simulink model "FlightSim"  (R2023a)
settings: Solver=VariableStepAuto  StartTime=0.0  StopTime=20  RelTol=1e-3
model workspace (MAT data in the model file):
  polyCoefficient = [1 1 1]
values from neighbouring files (symbols this model uses):
  airDensity = 1.2250    (= 1.225)    [FlightSimSetup.m]
  airKinematicViscosity = 1.48*10^-5    (= 1.48e-05)    [FlightSimSetup.m]
  AOA = deg2rad(0)    [FlightSimSetup.m]
  axialDistanceMACCGx = abs(finMACLeadingEdgeLocation) + finLeadingEdgeCGxlength    [finParameters.m]
  bodyDiameter = 2/100    (= 0.02)    [FlightSimSetup.m]
  bodyLength = 20/100    (= 0.2)    [FlightSimSetup.m]
  bodyLongitudinalInertia = bodyMass*( ((bodyDiameter/2).^2)/4 + ((bodyLength).^2)/12)    [InertiaParameters.m]
  bodyMass = 12/1000    (= 0.012)    [FlightSimSetup.m]
  bodyPlanformArea = bodyDiameter*bodyLength    (= 0.004)    [FlightSimSetup.m]
  bodyRoughness = 60*10^-6    (= 6e-05)    [FlightSimSetup.m]
  casingLongitudinalInertia = motorCasingMass/12*(3*(motorOuterRadius.^2+motorInnerRadius.^2)+motorLength.^2)    [InertiaParameters.m]
  cYaw = 0    [FlightSimSetup.m]
  dIdt = zeros(3)    [InertiaParameters.m]
  finArea = (finRootChord+finTailChord)*finSpan/2    [finParameters.m]
  finCantAngle = deg2rad(0)    [FlightSimSetup.m]
  finCentroidAxial = finSpan/3 * ( (finRootChord + 2*finTailChord)/(finRootChord + finTailChord) ) - backOffset    [finParameters.m]
  finCount = 4    [FlightSimSetup.m]
  finFrontSweepAngle = acos(dot(frontSweepAngleRefVector1, frontSweepAngleRefVector2) / (norm(frontSweepAngleRefVector1) * norm(frontSweepAngleRefVector2)))    [finParameters.m]
  finLeadingEdgeNoseConeDistance = bodyLength + noseConeHeight - finRootChord + backOffset    [finParameters.m]
  finLongitudinalInertia = finLongitudinalInertia * singleFinMass * finCount    [InertiaParameters.m]
  finMACLeadingEdgeLocation = abs( integral(finMACLeadingEdgeLocationFunction, 0, finSpan)/finArea )    [finParameters.m]
  finMACLength = integral(chordLengthFunction, 0, finSpan)/finArea    [finParameters.m]
  finMACNoseconeAxialDistance = finLeadingEdgeNoseConeDistance + finMACLeadingEdgeLocation+ 1/2*finMACLength    [finParameters.m]
  finMidChordSweepAngle = acos(dot(midChordAngleRefVector1, midChordAngleRefVector2) / (norm(midChordAngleRefVector1) * norm(midChordAngleRefVector2)))    [finParameters.m]
  finProfileType = 3    [FlightSimSetup.m]
  finRootChord = abs(P_frontRoot(2)-P_backRoot(2))    [finParameters.m]
  finSpan = abs(P_frontTrail(1)- P_frontRoot(1))    [finParameters.m]
  finTailChord = abs(P_frontTrail(2)-P_backTrail(2))    [finParameters.m]
  finThickness = 0.15/100    (= 0.0015)    [FlightSimSetup.m]
  finX_t = P_frontRoot(2) - P_frontTrail(2)    [finParameters.m]
  launchLugInnerDiameter = 0    [FlightSimSetup.m]
  launchLugLength = 0    [FlightSimSetup.m]
  launchLugOuterDiameter = 0    [FlightSimSetup.m]
  machNum = 0    [FlightSimSetup.m]
  motorCasingMass = motorInitialTotalMass - motorInitialPropMass    (= 0.071)    [FlightSimSetup.m]
  motorInitialPropMass = 83/1000    (= 0.083)    [FlightSimSetup.m]
  motorInnerRadius = motorOuterRadius - casingThickness    [InertiaParameters.m]
  motorLength = 156/1000    (= 0.156)    [FlightSimSetup.m]
  motorTotalImpulse = 129.9    [FlightSimSetup.m]
  noseConeHeight = 6/100    (= 0.06)    [FlightSimSetup.m]
  noseConeJoinAngle = pi/2 - atan(noseConeHeight/(bodyDiameter/2))    [FlightSimSetup.m]
  noseConeLongitudinalInertia = noseConeLongitudinalInertia*noseConeMass    [noseConeParameters.m]
  noseConeMass = 5/1000    (= 0.005)    [FlightSimSetup.m]
  noseConePlanformArea = (1/2)*bodyDiameter* noseConeHeight    [noseConeParameters.m]
  noseConeType = 8    [FlightSimSetup.m]
  noseConeVolume = (1/3)*noseConeHeight*(bodyDiameter/2)^2*pi    [noseConeParameters.m]
  noseConeWettedArea = pi*sqrt( (bodyDiameter/2)^2 + noseConeHeight^2)*bodyDiameter/2    [noseConeParameters.m]
  pitchCenterX = 0    [FlightSimSetup.m]
  pitchRate = 0    [FlightSimSetup.m]
  radialMACPosition = finMACSpanwisePosition + bodyDiameter/2    [finParameters.m]
  refArea = bodyDiameter^2*pi/4    (= 0.000314159265359)    [FlightSimSetup.m]
  rocketLength = (20+6)/100    (= 0.26)    [FlightSimSetup.m]
  rocketNonMotorMass = noseConeMass + bodyMass + totalFinMass    (= 0.021)    [FlightSimSetup.m]
  rocketRotationalInertia = bodyRotationalInertia + casingRotationalInertia + finRotationalInertia + noseConeLongitudinalInertia    [InertiaParameters.m]
  rocketVelocityMag = machNum*340.17    (= 0)    [FlightSimSetup.m]
  rollRate = 0    [FlightSimSetup.m]
  totalFinMass = singleFinMass * finCount    (= 0.004)    [FlightSimSetup.m]
  windAngle = 0    [FlightSimSetup.m]
  yawRate = 0    [FlightSimSetup.m]
libraries: shared6dof x1, aerolibanim x1, aerolibadyn x1, aerolibbdyn x1, sharedutil x1
(part 1 of 3)
settings Model: Creator=ericyang  LastModifiedBy=ericyang
settings DataIOCC: ExternalInput=[t,u]

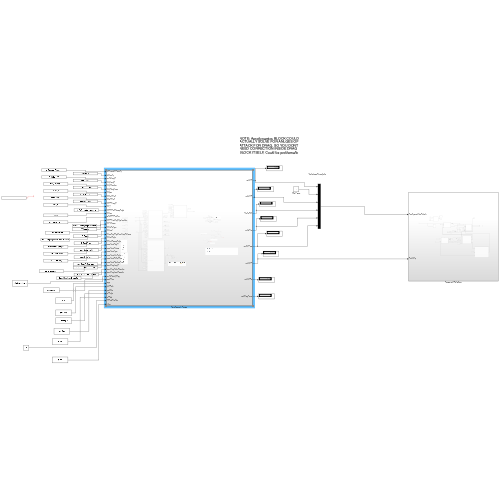
[thumbnail: Simulink's stored preview of the top-level diagram]
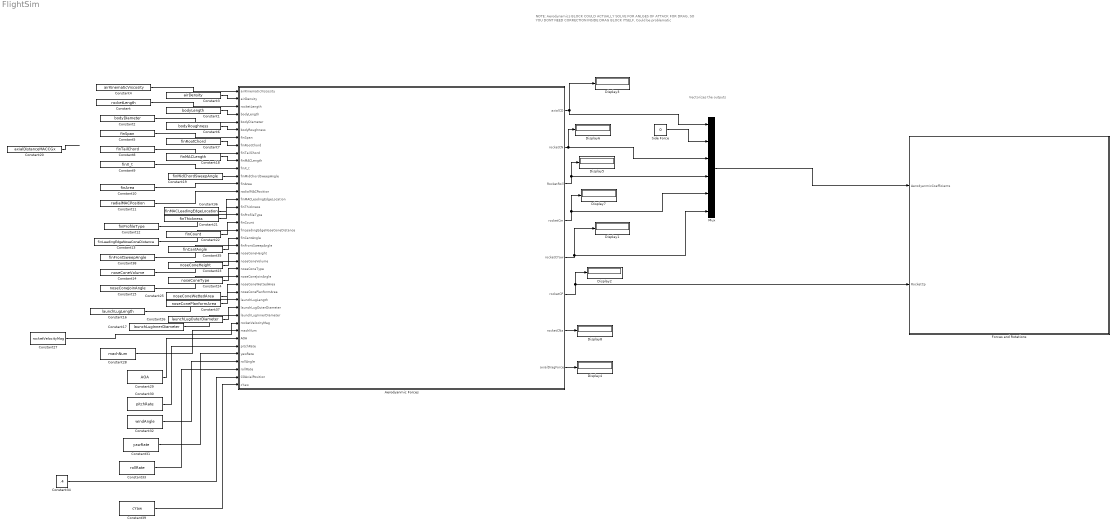
== system FlightSim ==
  note: NOTE: Aerodynamics BLOCK COULD ACTUALLY SOLVE FOR ANLGES OF ATTACK FOR DRAG, SO YOU DONT NEED CORRECTION INSIDE DRAG BLOCK ITSELF, Could be problematic
  note: Vectorizes the outputs
  Aerodyanmic Forces : Subsystem
  Constant : Constant  Value=rocketLength
  Constant1 : Constant  Value=bodyLength
  Constant10 : Constant  Value=finArea
  Constant11 : Constant  Value=radialMACPosition
  Constant12 : Constant  Value=finProfileType
  Constant13 : Constant  Value=finLeadingEdgeNoseConeDistance
  Constant14 : Constant  Value=noseConeVolume
  Constant15 : Constant  Value=noseConeJoinAngle
  Constant16 : Constant  Value=launchLugLength
  Constant17 : Constant  Value=launchLugInnerDiameter
  Constant18 : Constant  Value=finMACLength
  Constant19 : Constant  Value=finMidChordSweepAngle
  Constant2 : Constant  Value=bodyDiameter
  Constant20 : Constant  Value=axialDistanceMACCGx
  Constant21 : Constant  Value=finThickness
  Constant22 : Constant  Value=finCount
  Constant23 : Constant  Value=noseConeHeight
  Constant24 : Constant  Value=noseConeType
  Constant25 : Constant  Value=noseConeWettedArea
  Constant26 : Constant  Value=launchLugOuterDiameter
  Constant27 : Constant  Value=rocketVelocityMag
  Constant28 : Constant  Value=machNum
  Constant29 : Constant  Value=AOA
  Constant3 : Constant  Value=airDensity
  Constant30 : Constant  Value=pitchRate
  Constant31 : Constant  Value=yawRate
  Constant32 : Constant  Value=windAngle
  Constant33 : Constant  Value=rollRate
  Constant34 : Constant  Value=.4
  Constant35 : Constant  Value=finCantAngle
  Constant36 : Constant  Value=finMACLeadingEdgeLocation
  Constant37 : Constant  Value=noseConePlanformArea
  Constant38 : Constant  Value=finFrontSweepAngle
  Constant39 : Constant  Value=cYaw
  Constant4 : Constant  Value=airKinematicViscosity
  Constant5 : Constant  Value=finSpan
  Constant6 : Constant  Value=bodyRoughness
  Constant7 : Constant  Value=finRootChord
  Constant8 : Constant  Value=finTailChord
  Constant9 : Constant  Value=finX_t
  Display{1..8} (8 alike) : Display
  Forces and Rotations : Subsystem
  Mux : Mux  Inputs=6
  Side Force : Constant  Value=0
  wires:
  Constant/1 -> Aerodyanmic Forces/3(rocketLength)
  Constant1/1 -> Aerodyanmic Forces/4(bodyLength)
  Constant2/1 -> Aerodyanmic Forces/5(bodyDiameter)
  Constant4/1 -> Aerodyanmic Forces/1(airKinematicViscosity)
  Constant3/1 -> Aerodyanmic Forces/2(airDensity)
  Constant6/1 -> Aerodyanmic Forces/6(bodyRoughness)
  Constant5/1 -> Aerodyanmic Forces/7(finSpan)
  Constant7/1 -> Aerodyanmic Forces/8(finRootChord)
  Constant8/1 -> Aerodyanmic Forces/9(finTailChord)
  Constant9/1 -> Aerodyanmic Forces/11(finX_t)
  Constant10/1 -> Aerodyanmic Forces/13(finArea)
  Constant11/1 -> Aerodyanmic Forces/14(radialMACPosition)
  Constant12/1 -> Aerodyanmic Forces/17(finProfileType)
  Constant13/1 -> Aerodyanmic Forces/19(finLeadingEdgeNoseConeDistance)
  Constant14/1 -> Aerodyanmic Forces/23(noseConeVolume)
  Constant15/1 -> Aerodyanmic Forces/25(noseConeJoinAngle)
  Constant16/1 -> Aerodyanmic Forces/28(launchLugLength)
  Constant17/1 -> Aerodyanmic Forces/30(launchLugInnerDiameter)
  Constant18/1 -> Aerodyanmic Forces/10(finMACLength)
  Constant19/1 -> Aerodyanmic Forces/12(finMidChordSweepAngle)
  Constant20/1 -> ?
  Constant21/1 -> Aerodyanmic Forces/16(finThickness)
  Constant22/1 -> Aerodyanmic Forces/18(finCount)
  Constant23/1 -> Aerodyanmic Forces/22(noseConeHeight)
  Constant24/1 -> Aerodyanmic Forces/24(noseConeType)
  Constant25/1 -> Aerodyanmic Forces/26(noseConeWettedArea)
  Constant26/1 -> Aerodyanmic Forces/29(launchLugOuterDiameter)
  Constant27/1 -> Aerodyanmic Forces/31(rocketVelocityMag)
  Constant28/1 -> Aerodyanmic Forces/32(machNum)
  Constant29/1 -> Aerodyanmic Forces/33(AOA)
  Constant30/1 -> Aerodyanmic Forces/34(pitchRate)
  Constant31/1 -> Aerodyanmic Forces/35(yawRate)
  Constant32/1 -> Aerodyanmic Forces/36(rollAngle)
  Constant33/1 -> Aerodyanmic Forces/37(rollRate)
  Aerodyanmic Forces/6(rocketCP) -> Forces and Rotations/2(RocketCp), Display2/1
  Aerodyanmic Forces/1(axialCD) -> Display3/1, Mux/1
  Aerodyanmic Forces/8(axialDragForce) -> Display4/1
  Aerodyanmic Forces/3(RocketRoll) -> Mux/4, Display5/1
  Aerodyanmic Forces/2(rocketCN) -> Display6/1, Mux/3
  Aerodyanmic Forces/4(rocketCm) -> Mux/5, Display7/1
  Constant35/1 -> Aerodyanmic Forces/20(finCantAngle)
  Constant36/1 -> Aerodyanmic Forces/15(finMACLeadingEdgeLocation)
  Constant37/1 -> Aerodyanmic Forces/27(noseConePlanformArea)
  Constant38/1 -> Aerodyanmic Forces/21(finFrontSweepAngle)
  Constant39/1 -> Aerodyanmic Forces/39(cYaw)
  Aerodyanmic Forces/7(rocketCNa) -> Display8/1
  Side Force/1 -> Mux/2
  Aerodyanmic Forces/5(rocketCYaw) -> Mux/6, Display1/1
  Mux/1 -> Forces and Rotations/1(AerodyanmicCoefficients)
  Constant34/1 -> Aerodyanmic Forces/38(CGAxialPosition)

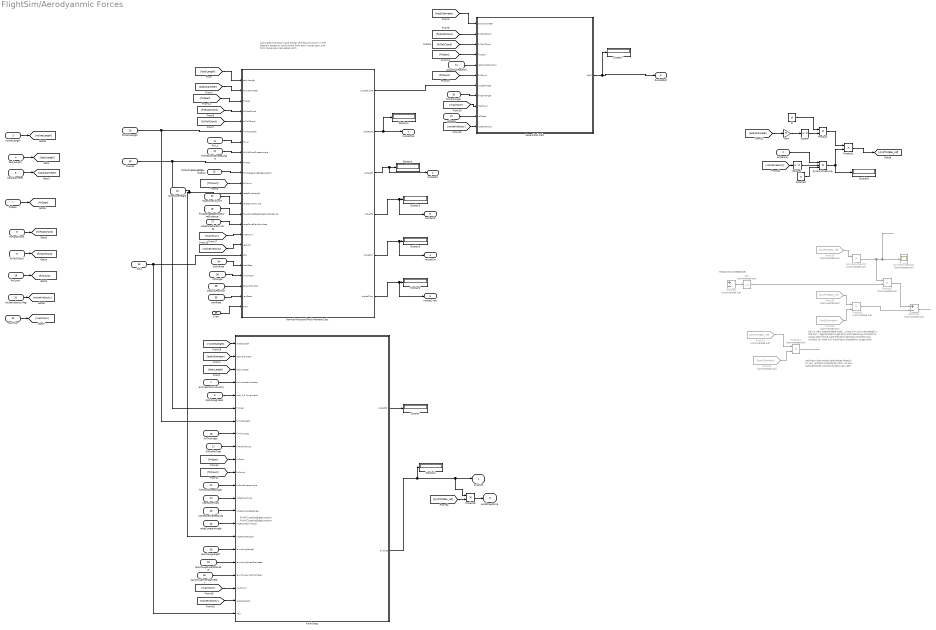
== system FlightSim/Aerodyanmic Forces ==
  note: finMACLeadingEdgeLocation
  note: finMACLeadingEdgeLocation
  note: Relative to NOSECONE
  note: nothing in the rocket contributes directly to yaw (without canards for now), so only outside forces would provide a yaw rate
  note: this is very questionable math... since Cm isn't calculated in the sim, i figured that to get form pitch damping moment to actual pitch force, take the pitch damping moment and multiply by what Cm would be multiplied by to get pitch
  note: calculates the total N and Center of Pressure shown in the diagram based on contriutions from each rocket part, and from those also calculates pitch
  airKinematicViscosity : Inport
  airDensity : Inport  Port=2
  rocketLength : Inport  Port=3
  bodyLength : Inport  Port=4
  bodyDiameter : Inport  Port=5
  bodyRoughness : Inport  Port=6
  finSpan : Inport  Port=7
  finRootChord : Inport  Port=8
  finTailChord : Inport  Port=9
  finMACLength : Inport  Port=10
  finX_t : Inport  Port=11
  finMidChordSweepAngle : Inport  Port=12
  finArea : Inport  Port=13
  radialMACPosition : Inport  Port=14
  finMACLeadingEdgeLocation : Inport  Port=15
  finThickness : Inport  Port=16
  finProfileType : Inport  Port=17
  finCount : Inport  Port=18
  finLeadingEdgeNoseConeDistance : Inport  Port=19
  finCantAngle : Inport  Port=20
  finFrontSweepAngle : Inport  Port=21
  noseConeHeight : Inport  Port=22
  noseConeVolume : Inport  Port=23
  noseConeType : Inport  Port=24
  noseConeJoinAngle : Inport  Port=25
  noseConeWettedArea : Inport  Port=26
  noseConePlanformArea : Inport  Port=27
  launchLugLength : Inport  Port=28
  launchLugOuterDiameter : Inport  Port=29
  launchLugInnerDiameter : Inport  Port=30
  rocketVelocityMag : Inport  Port=31
  machNum : Inport  Port=32
  AOA : Inport  Port=33
  pitchRate : Inport  Port=34
  yawRate : Inport  Port=35
  rollAngle : Inport  Port=36
  rollRate : Inport  Port=37
  CGAxialPosition : Inport  Port=38
  cYaw : Inport  Port=39
  Abs : Abs  Commented=on  SaturateOnIntegerOverflow=off
  Axial Drag : Subsystem
  Canard Roll Calc : Subsystem
  Constant : Constant  Value=.5
  Display{,1..8} (9 alike) : Display
  From23 : From  Commented=on  GotoTag=bodyDiameter
  From24 : From  Commented=on  GotoTag=bodyDiameter
  From28 : From  Commented=on  GotoTag=dynPTIMESA_ref
  From30 : From  Commented=on  GotoTag=dynPTIMESA_ref
  From31 : From  Commented=on  GotoTag=dynPTIMESA_ref
  Gain : Gain  Gain=.5
  Normal Force and Pitch Moment Calc : Subsystem
  Pi : Constant  Value=pi  OutDataTypeStr=Inherit: Inherit via back propagation
  Product : Product
  Product1 : Product
  Product2 : Product  Commented=on
  Product3 : Product  Commented=on  Inputs=3
  Product4 : Product  Commented=on  Inputs=3
  Product6 : Product
  Square : Math  Operator=square
  Square1 : Math  Operator=square
  Subtract : Sum  Commented=on  Inputs=+-
  Subtract1 : Sum  Commented=on  Inputs=+-
  dynamicPressure : Product  Inputs=3
  normalForceAtCP : Product  Commented=on
  normalForceScope : Scope  Commented=on
  axialCD : Outport
  rocketCN : Outport  Port=2
  RocketRoll : Outport  Port=3
  rocketCm : Outport  Port=4
  rocketCYaw : Outport  Port=5
  rocketCP : Outport  Port=6
  rocketCNa : Outport  Port=7
  axialDragForce : Outport  Port=8
  wires:
  finX_t/1 -> Normal Force and Pitch Moment Calc/7(finX_t)
  finMidChordSweepAngle/1 -> Normal Force and Pitch Moment Calc/8(finMidChordSweepAngle)
  finArea/1 -> Axial Drag/6(finArea), Normal Force and Pitch Moment Calc/9(finArea)
  finProfileType/1 -> Axial Drag/9(finProfileType)
  finThickness/1 -> Axial Drag/8(finThickness)
  radialMACPosition/1 -> Canard Roll Calc/5(radialMACPosition)
  finLeadingEdgeNoseConeDistance/1 -> Normal Force and Pitch Moment Calc/14(finLeadingEdgeNoseConeDistance)
  noseConeType/1 -> Axial Drag/13(noseConeType)
  noseConeWettedArea/1 -> Axial Drag/14(noseConeWettedArea)
  noseConeJoinAngle/1 -> Axial Drag/15(noseConeJoinAngle)
  noseConeVolume/1 -> Normal Force and Pitch Moment Calc/13(noseConeVolume)
  launchLugLength/1 -> Axial Drag/17(launchLugLength)
  launchLugOuterDiameter/1 -> Axial Drag/18(launchLugOuterDiameter)
  launchLugInnerDiameter/1 -> Axial Drag/19(launchLugInnerDiameter)
  rollRate/1 -> Canard Roll Calc/10(" rollRate")
  Normal Force and Pitch Moment Calc/1(singleFinCNa) -> Canard Roll Calc/7(singleFinCNa)
  bodyLength/1 -> [bodyLength]
  bodyDiameter/1 -> [bodyDiameter]
  finCount/1 -> [finCount]
  [bodyLength] -> Normal Force and Pitch Moment Calc/1(bodyLength)
  [bodyLength] -> Axial Drag/3(bodyLength)
  [bodyDiameter] -> Normal Force and Pitch Moment Calc/2(bodyDiameter)
  [bodyDiameter] -> Canard Roll Calc/1(bodyDiameter)
  [bodyDiameter] -> Axial Drag/2(bodyDiameter)
  airKinematicViscosity/1 -> Axial Drag/4(airKinematicViscocity)
  bodyRoughness/1 -> Axial Drag/5(bodyR_S (roughness))
  finRootChord/1 -> [finRootChord]
  finTailChord/1 -> [finTailChord]
  [finRootChord] -> Normal Force and Pitch Moment Calc/4(finRootChord)
  [finRootChord] -> Canard Roll Calc/2(finRootChord)
  [finTailChord] -> Normal Force and Pitch Moment Calc/5(finTailChord)
  [finTailChord] -> Canard Roll Calc/3(finTailChord)
  [finCount] -> Normal Force and Pitch Moment Calc/11(finCount)
  [finCount] -> Canard Roll Calc/6(finCount)
  [finCount] -> Axial Drag/11(finCount)
  finSpan/1 -> [finSpan]
  [finSpan] -> Normal Force and Pitch Moment Calc/3(finSpan)
  [finSpan] -> Canard Roll Calc/4(finSpan)
  [finSpan] -> Axial Drag/10(finSpan)
  finMACLength/1 -> Axial Drag/7(finMACLength), Normal Force and Pitch Moment Calc/6(finMACLength)
  AOA/1 -> Normal Force and Pitch Moment Calc/18(AOA), Axial Drag/22(AOA)
  pitchRate/1 -> Normal Force and Pitch Moment Calc/19(pitchRate)
  rocketVelocityMag/1 -> [rocketVelocity]
  [rocketVelocity] -> Normal Force and Pitch Moment Calc/17(velocity)
  [rocketVelocity] -> Axial Drag/21(rocketVelocity)
  rollAngle/1 -> Normal Force and Pitch Moment Calc/20(windAngle)
  machNum/1 -> [machNum]
  [machNum] -> Normal Force and Pitch Moment Calc/16(machNum)
  [machNum] -> Axial Drag/20(machNum)
  [rocketVelocity] -> Canard Roll Calc/11(" rocketVelocity")
  [machNum] -> Canard Roll Calc/9(" MachNum")
  [bodyDiameter] -> Gain/1
  Gain/1 -> Square/1
  Square/1 -> Product/2
  Pi/1 -> Product/1
  [rocketVelocity] -> Square1/1
  airDensity/1 -> dynamicPressure/1
  Square1/1 -> dynamicPressure/2
  Constant/1 -> dynamicPressure/3
  Product/1 -> Product1/1
  dynamicPressure/1 -> Product1/2, Display8/1
  Product1/1 -> [dynPTIMESA_ref]
  Normal Force and Pitch Moment Calc/3(rocketCN) -> rocketCN/1, Display1/1
  normalForceAtCP/1 -> Product2/1, normalForceScope/1
  Normal Force and Pitch Moment Calc/4(rocketCp) -> rocketCP/1, Display3/1
  Subtract/1 -> Abs/1
  Abs/1 -> Product2/2
  Product2/1 -> Subtract1/1
  From23/1 -> Product3/3
  From24/1 -> Product4/3
  Product3/1 -> Subtract1/2
  Subtract1/1 -> ?
  Product4/1 -> ?
  Axial Drag/2(axialCD) -> Display2/1, Product6/1, axialCD/1
  Canard Roll Calc/1(cRoll) -> RocketRoll/1, Display7/1
  rocketLength/1 -> [rocketLength]
  [rocketLength] -> Axial Drag/1(rocketLength)
  CGAxialPosition/1 -> Normal Force and Pitch Moment Calc/21(CGAxialPositon)
  From28/1 -> normalForceAtCP/1
  From30/1 -> Product3/1
  From31/1 -> Product4/1
  [dynPTIMESA_ref] -> Product6/2
  Product6/1 -> axialDragForce/1
  yawRate/1 -> Normal Force and Pitch Moment Calc/22(yawRate)
  finCantAngle/1 -> Canard Roll Calc/8(finCantAngle)
  finMACLeadingEdgeLocation/1 -> Normal Force and Pitch Moment Calc/10(finMACLeadingEdgeLocation)
  Axial Drag/1(rocketCD) -> Display/1
  Normal Force and Pitch Moment Calc/2(rocketCna) -> rocketCNa/1, Display4/1
  noseConePlanformArea/1 -> Normal Force and Pitch Moment Calc/15(noseConePlanformArea)
  finFrontSweepAngle/1 -> Axial Drag/12(finFrontSweepAngle)
  noseConeHeight/1 -> Normal Force and Pitch Moment Calc/12(noseConeHeight), Axial Drag/16(noseConeHeight)
  cYaw/1 -> Normal Force and Pitch Moment Calc/23(cYaw)
  Normal Force and Pitch Moment Calc/5(rocketCm) -> rocketCm/1, Display5/1
  Normal Force and Pitch Moment Calc/6(rocketCYaw) -> rocketCYaw/1, Display6/1

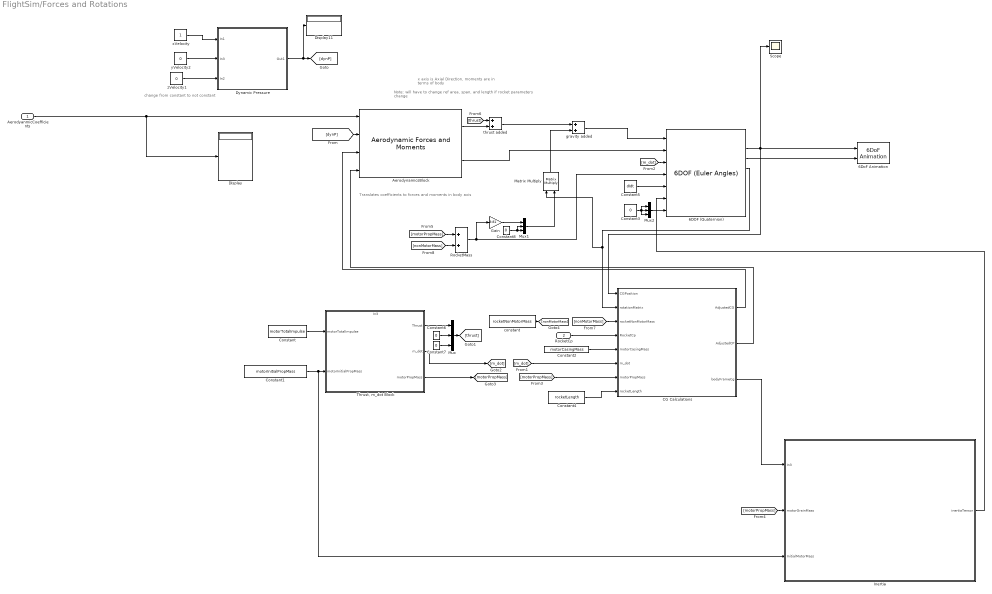
== system FlightSim/Forces and Rotations ==
  note: Translates coefficients to forces and moments in body axis
  note: x axis is Axial Direction, moments are
      | in terms of body
  note: Note: will have to change ref area, span, and length if rocket parameters change
  note: change from constant to not constant
  AerodyanmicCoefficients : Inport
  RocketCp : Inport  Port=2
  6DOF (Quaternion) : shared6dof/6DOF (Euler Angles)  units=Metric (MKS)  mtype=Custom Variable  rep=Quaternion  xme_0=[0.08148 0 0]  Vm_0=[0 0 0]  eul_0=[0 0 0]  pm_0=[0 0 0]  mass_0=1.0  mass_e=0.5  mass_f=2.0  inertia=eye(3)  inertia_e=eye(3)  inertia_f=2*eye(3)  k_quat=1.0  vre_flag=on  abi_flag=off  aMode=0  xme_statename=''  Vm_statename=''  eul_statename=''  quat_statename=''  pm_statename=''  mass_statename=''
      settings: LibrarySourceBlock=aerolib6dof2/6DOF (Quaternion)
  6DoF Animation : aerolibanim/6DoF Animation  u1=[-2000 2000 -2000 2000 -5000 5000]  u2=0.1  u3=200  u4=[4000 0 -5000]  u5=Fly alongside  u6=[5000 6000 -8000]  u7=10  u8=on
  AerodynamicsBlock : aerolibadyn/Aerodynamic Forces and Moments   inputAxes=Body  outputForceAxes=Body  outputMomentAxes=Body  S=0.00031416  b=0.02  cbar=0.02
  CG Calculations : Subsystem
  Constant : Constant  Value=motorTotalImpulse
  Constant1 : Constant  Value=motorInitialPropMass
  Constant2 : Constant  Value=motorCasingMass
  Constant{3,6..8} (4 alike) : Constant  Value=0
  Constant4 : Constant  Value=rocketLength
  Constant5 : Constant  Value=dIdt
  Display : Display
  Display11 : Display
  Dynamic Pressure : Subsystem
  Gain : Gain  Gain=9.81
  Inertia : Subsystem
  Matrix Multiply : Product  Multiplication=Matrix(*)
  Mux : Mux  Inputs=3
  Mux1 : Mux  Inputs=3
  Mux2 : Mux  Inputs=3
  RocketMass : Sum
  Scope : Scope
  "Thrust, m_dot Block" : Subsystem
      settings: PortSchema="{\"entries\":[{\"content\":{\"sides\":[{\"content\":{\"connectorIds\":[\"In1\",\"In2\"]},\"type\":\"ConnectorPlacement.EquallySpacedRectSide\",\"uuid\":\"f0970325-3972-4de5-b8ef-50cf81fedc12\"},{\"content\":{\"connectorIds\":[\"Out1\",\"Out2\",\"Out3\"],\"side\":\"RIGHT\"},\"type\":\"ConnectorPlacement.EquallySpacedRectSide\",\"uuid\":\"1b7266ef-425e-40c5-9751-51bd11785909\"},{\"content\":{\"connectorIds\":[\"In3\"],\"side\":\"TOP\"},\"type\":\"ConnectorPlacement.EquallySpacedRectSide\",\"uuid\":\"ded2c137-74ff-4527-8529-fcff9e0b98b8\"}]},\"type\":\"ConnectorPlacement.EquallySpacedConnector\",\"uuid\":\"51f8d04c-b243-453f-aa0e-d7febef3be8d\"}],\"packageUris\":[\"http://schema.mathworks.com/mf0/Connector/R2020b\"],\"version\":\"1.0\"}"
  constant : Constant  Value=rocketNonMotorMass
  gravity added : Sum
  thrust added : Sum
  xVelocity : Constant
  yVelocity2 : Constant  Value=0
  zVelocity1 : Constant  Value=0
  wires:
  6DOF (Quaternion)/3 -> 6DoF Animation/2
  6DOF (Quaternion)/2 -> CG Calculations/1(CGPosition), 6DoF Animation/1, Scope/1
  [dynP] -> AerodynamicsBlock/2
  AerodyanmicCoefficients/1 -> Display/1, AerodynamicsBlock/1
  Dynamic Pressure/1(Out1) -> Display11/1, [dynP]
  yVelocity2/1 -> Dynamic Pressure/2(In3)
  zVelocity1/1 -> Dynamic Pressure/3(In2)
  xVelocity/1 -> Dynamic Pressure/1(In1)
  Constant1/1 -> Inertia/3(InitialMotorMass), "Thrust, m_dot Block"/2(motorInitialPropMass)
  Constant/1 -> "Thrust, m_dot Block"/1(motorTotalImpulse)
  Constant2/1 -> CG Calculations/5(motorCasingMass)
  6DOF (Quaternion)/4 -> Matrix Multiply/1, CG Calculations/2(rotationMatrix)
  Constant4/1 -> CG Calculations/8(rocketLength)
  RocketCp/1 -> CG Calculations/4(RocketCp)
  CG Calculations/1(AdjustedCG) -> AerodynamicsBlock/3
  CG Calculations/2(AdjustedCP) -> AerodynamicsBlock/4
  AerodynamicsBlock/1 -> thrust added/2
  CG Calculations/3(bodyFrameCg) -> Inertia/1(In3)
  Inertia/1(inertiaTensor) -> 6DOF (Quaternion)/6
  Constant5/1 -> 6DOF (Quaternion)/5
  RocketMass/1 -> Gain/1, 6DOF (Quaternion)/4
  "Thrust, m_dot Block"/2(m_dot) -> [m_dot]
  [m_dot] -> CG Calculations/6(m_dot)
  [m_dot] -> 6DOF (Quaternion)/3
  "Thrust, m_dot Block"/3(motorPropMass) -> [motorPropMass]
  [motorPropMass] -> CG Calculations/7(motorPropMass)
  [motorPropMass] -> Inertia/2(motorGrainMass)
  [motorPropMass] -> RocketMass/1
  Constant6/1 -> Mux/2
  Constant7/1 -> Mux/3
  "Thrust, m_dot Block"/1(Thrust) -> Mux/1
  Mux/1 -> [thrust]
  [thrust] -> thrust added/1
  constant/1 -> [nonMotorMass]
  [nonMotorMass] -> CG Calculations/3(rocketNonMotorMass)
  [nonMotorMass] -> RocketMass/2
  Mux1/1 -> Matrix Multiply/2
  Matrix Multiply/1 -> gravity added/2
  thrust added/1 -> gravity added/1
  gravity added/1 -> 6DOF (Quaternion)/1
  Gain/1 -> Mux1/1
  Constant8/1 -> Mux1/2, Mux1/3
  Constant3/1 -> Mux2/3, Mux2/1, Mux2/2
  Mux2/1 -> 6DOF (Quaternion)/7
  AerodynamicsBlock/2 -> 6DOF (Quaternion)/2

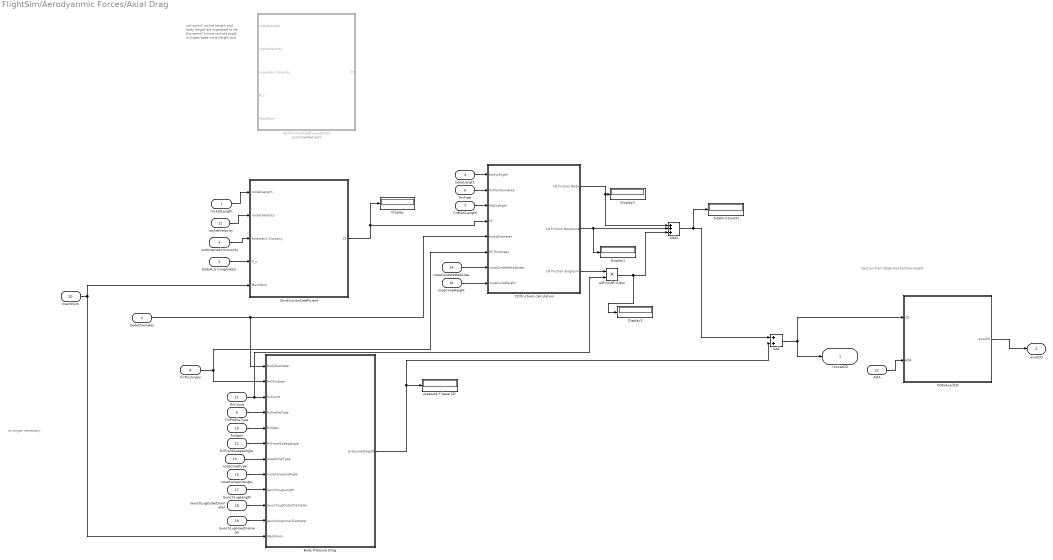
== system FlightSim/Aerodyanmic Forces/Axial Drag ==
  note: no longer necessary
  note: not sure if rocket length and body lenght are supposed to be the same? (since rocketLength includes nose cone height too)
  note: Section from Open Rocket Document
  rocketLength : Inport
  bodyDiameter : Inport  Port=2
  bodyLength : Inport  Port=3
  airKinematicViscocity : Inport  Port=4
  bodyR_S (roughness) : Inport  Port=5
  finArea : Inport  Port=6
  finMACLength : Inport  Port=7
  finThickness : Inport  Port=8
  finProfileType : Inport  Port=9
  finSpan : Inport  Port=10
  finCount : Inport  Port=11
  finFrontSweepAngle : Inport  Port=12
  noseConeType : Inport  Port=13
  noseConeWettedArea : Inport  Port=14
  noseConeJoinAngle : Inport  Port=15
  noseConeHeight : Inport  Port=16
  launchLugLength : Inport  Port=17
  launchLugOuterDiameter : Inport  Port=18
  launchLugInnerDiameter : Inport  Port=19
  machNum : Inport  Port=20
  rocketVelocity : Inport  Port=21
  AOA : Inport  Port=22
  Add : Sum
  Add1 : Sum  Inputs=+++
  Body Pressure Drag : Subsystem
  CD(friction) calculation : Subsystem
  CDtoAxailCD : Subsystem
  Display{,1..3} (4 alike) : Display
  SkinFrictionCoefficeint(OLD) : Subsystem  Commented=on
  SkinFrictionCoefficient : Subsystem
  allFinCdFriciton : Product
  pressure + base CD : Display
  totalFrictionCD : Display
  rocketCD : Outport
  axialCD : Outport  Port=2
  wires:
  CD(friction) calculation/1(Cd Friction Body) -> Add1/1, Display2/1
  Body Pressure Drag/1(pressureDragCD) -> Add/2, pressure + base CD/1
  Add/1 -> rocketCD/1, CDtoAxailCD/1(CD)
  CDtoAxailCD/1(axialCD) -> axialCD/1
  bodyDiameter/1 -> Body Pressure Drag/1(bodyDiameter), CD(friction) calculation/5(bodyDiameter)